simulink model "jgowans_fft_4chan"  (R2012b) (continued)
== system jgowans_fft_4chan/cross_multiplier1 == (continued)
      mask init:
      | pipeline_init(gcb,'latency',latency);
      settings: AttributesFormatString=Latency = %<latency>
      mask Display:
      | port_label('input', 1, 'd');
      | port_label('output', 1, 'q');
      | disp(['Z^-', num2str(latency)]);
  pipeline0_1_1_3 : Subsystem  AncestorBlock=casper_library_delays/pipeline  mask(pipeline){latency=fanout_latency}
      mask help:
      | An explicitly laid-out delay line to avoid mapping to SRL16s
      | for use in pipelining to help achieve timing closure.
      mask init:
      | pipeline_init(gcb,'latency',latency);
      settings: AttributesFormatString=Latency = %<latency>
      mask Display:
      | port_label('input', 1, 'd');
      | port_label('output', 1, 'q');
      | disp(['Z^-', num2str(latency)]);
  pipeline0_2_0_0 : Subsystem  AncestorBlock=casper_library_delays/pipeline  mask(pipeline){latency=fanout_latency}
      mask help:
      | An explicitly laid-out delay line to avoid mapping to SRL16s
      | for use in pipelining to help achieve timing closure.
      mask init:
      | pipeline_init(gcb,'latency',latency);
      settings: AttributesFormatString=Latency = %<latency>
      mask Display:
      | port_label('input', 1, 'd');
      | port_label('output', 1, 'q');
      | disp(['Z^-', num2str(latency)]);
  pipeline0_2_0_1 : Subsystem  AncestorBlock=casper_library_delays/pipeline  mask(pipeline){latency=fanout_latency}
      mask help:
      | An explicitly laid-out delay line to avoid mapping to SRL16s
      | for use in pipelining to help achieve timing closure.
      mask init:
      | pipeline_init(gcb,'latency',latency);
      settings: AttributesFormatString=Latency = %<latency>
      mask Display:
      | port_label('input', 1, 'd');
      | port_label('output', 1, 'q');
      | disp(['Z^-', num2str(latency)]);
  pipeline0_2_0_2 : Subsystem  AncestorBlock=casper_library_delays/pipeline  mask(pipeline){latency=fanout_latency}
      mask help:
      | An explicitly laid-out delay line to avoid mapping to SRL16s
      | for use in pipelining to help achieve timing closure.
      mask init:
      | pipeline_init(gcb,'latency',latency);
      settings: AttributesFormatString=Latency = %<latency>
      mask Display:
      | port_label('input', 1, 'd');
      | port_label('output', 1, 'q');
      | disp(['Z^-', num2str(latency)]);
  pipeline0_2_0_3 : Subsystem  AncestorBlock=casper_library_delays/pipeline  mask(pipeline){latency=fanout_latency}
      mask help:
      | An explicitly laid-out delay line to avoid mapping to SRL16s
      | for use in pipelining to help achieve timing closure.
      mask init:
      | pipeline_init(gcb,'latency',latency);
      settings: AttributesFormatString=Latency = %<latency>
      mask Display:
      | port_label('input', 1, 'd');
      | port_label('output', 1, 'q');
      | disp(['Z^-', num2str(latency)]);
  pipeline0_2_1_0 : Subsystem  AncestorBlock=casper_library_delays/pipeline  mask(pipeline){latency=fanout_latency}
      mask help:
      | An explicitly laid-out delay line to avoid mapping to SRL16s
      | for use in pipelining to help achieve timing closure.
      mask init:
      | pipeline_init(gcb,'latency',latency);
      settings: AttributesFormatString=Latency = %<latency>
      mask Display:
      | port_label('input', 1, 'd');
      | port_label('output', 1, 'q');
      | disp(['Z^-', num2str(latency)]);
  pipeline0_2_1_1 : Subsystem  AncestorBlock=casper_library_delays/pipeline  mask(pipeline){latency=fanout_latency}
      mask help:
      | An explicitly laid-out delay line to avoid mapping to SRL16s
      | for use in pipelining to help achieve timing closure.
      mask init:
      | pipeline_init(gcb,'latency',latency);
      settings: AttributesFormatString=Latency = %<latency>
      mask Display:
      | port_label('input', 1, 'd');
      | port_label('output', 1, 'q');
      | disp(['Z^-', num2str(latency)]);
  pipeline0_2_1_2 : Subsystem  AncestorBlock=casper_library_delays/pipeline  mask(pipeline){latency=fanout_latency}
      mask help:
      | An explicitly laid-out delay line to avoid mapping to SRL16s
      | for use in pipelining to help achieve timing closure.
      mask init:
      | pipeline_init(gcb,'latency',latency);
      settings: AttributesFormatString=Latency = %<latency>
      mask Display:
      | port_label('input', 1, 'd');
      | port_label('output', 1, 'q');
      | disp(['Z^-', num2str(latency)]);
  pipeline0_2_1_3 : Subsystem  AncestorBlock=casper_library_delays/pipeline  mask(pipeline){latency=fanout_latency}
      mask help:
      | An explicitly laid-out delay line to avoid mapping to SRL16s
      | for use in pipelining to help achieve timing closure.
      mask init:
      | pipeline_init(gcb,'latency',latency);
      settings: AttributesFormatString=Latency = %<latency>
      mask Display:
      | port_label('input', 1, 'd');
      | port_label('output', 1, 'q');
      | disp(['Z^-', num2str(latency)]);
  pipeline0_3_0_0 : Subsystem  AncestorBlock=casper_library_delays/pipeline  mask(pipeline){latency=fanout_latency}
      mask help:
      | An explicitly laid-out delay line to avoid mapping to SRL16s
      | for use in pipelining to help achieve timing closure.
      mask init:
      | pipeline_init(gcb,'latency',latency);
      settings: AttributesFormatString=Latency = %<latency>
      mask Display:
      | port_label('input', 1, 'd');
      | port_label('output', 1, 'q');
      | disp(['Z^-', num2str(latency)]);
  pipeline0_3_0_1 : Subsystem  AncestorBlock=casper_library_delays/pipeline  mask(pipeline){latency=fanout_latency}
      mask help:
      | An explicitly laid-out delay line to avoid mapping to SRL16s
      | for use in pipelining to help achieve timing closure.
      mask init:
      | pipeline_init(gcb,'latency',latency);
      settings: AttributesFormatString=Latency = %<latency>
      mask Display:
      | port_label('input', 1, 'd');
      | port_label('output', 1, 'q');
      | disp(['Z^-', num2str(latency)]);
  pipeline0_3_0_2 : Subsystem  AncestorBlock=casper_library_delays/pipeline  mask(pipeline){latency=fanout_latency}
      mask help:
      | An explicitly laid-out delay line to avoid mapping to SRL16s
      | for use in pipelining to help achieve timing closure.
      mask init:
      | pipeline_init(gcb,'latency',latency);
      settings: AttributesFormatString=Latency = %<latency>
      mask Display:
      | port_label('input', 1, 'd');
      | port_label('output', 1, 'q');
      | disp(['Z^-', num2str(latency)]);
  pipeline0_3_0_3 : Subsystem  AncestorBlock=casper_library_delays/pipeline  mask(pipeline){latency=fanout_latency}
      mask help:
      | An explicitly laid-out delay line to avoid mapping to SRL16s
      | for use in pipelining to help achieve timing closure.
      mask init:
      | pipeline_init(gcb,'latency',latency);
      settings: AttributesFormatString=Latency = %<latency>
      mask Display:
      | port_label('input', 1, 'd');
      | port_label('output', 1, 'q');
      | disp(['Z^-', num2str(latency)]);
  pipeline0_3_1_0 : Subsystem  AncestorBlock=casper_library_delays/pipeline  mask(pipeline){latency=fanout_latency}
      mask help:
      | An explicitly laid-out delay line to avoid mapping to SRL16s
      | for use in pipelining to help achieve timing closure.
      mask init:
      | pipeline_init(gcb,'latency',latency);
      settings: AttributesFormatString=Latency = %<latency>
      mask Display:
      | port_label('input', 1, 'd');
      | port_label('output', 1, 'q');
      | disp(['Z^-', num2str(latency)]);
  pipeline0_3_1_1 : Subsystem  AncestorBlock=casper_library_delays/pipeline  mask(pipeline){latency=fanout_latency}
      mask help:
      | An explicitly laid-out delay line to avoid mapping to SRL16s
      | for use in pipelining to help achieve timing closure.
      mask init:
      | pipeline_init(gcb,'latency',latency);
      settings: AttributesFormatString=Latency = %<latency>
      mask Display:
      | port_label('input', 1, 'd');
      | port_label('output', 1, 'q');
      | disp(['Z^-', num2str(latency)]);
  pipeline0_3_1_2 : Subsystem  AncestorBlock=casper_library_delays/pipeline  mask(pipeline){latency=fanout_latency}
      mask help:
      | An explicitly laid-out delay line to avoid mapping to SRL16s
      | for use in pipelining to help achieve timing closure.
      mask init:
      | pipeline_init(gcb,'latency',latency);
      settings: AttributesFormatString=Latency = %<latency>
      mask Display:
      | port_label('input', 1, 'd');
      | port_label('output', 1, 'q');
      | disp(['Z^-', num2str(latency)]);
  pipeline0_3_1_3 : Subsystem  AncestorBlock=casper_library_delays/pipeline  mask(pipeline){latency=fanout_latency}
      mask help:
      | An explicitly laid-out delay line to avoid mapping to SRL16s
      | for use in pipelining to help achieve timing closure.
      mask init:
      | pipeline_init(gcb,'latency',latency);
      settings: AttributesFormatString=Latency = %<latency>
      mask Display:
      | port_label('input', 1, 'd');
      | port_label('output', 1, 'q');
      | disp(['Z^-', num2str(latency)]);
  pipeline1_0_0_0 : Subsystem  AncestorBlock=casper_library_delays/pipeline  mask(pipeline){latency=fanout_latency}
      mask help:
      | An explicitly laid-out delay line to avoid mapping to SRL16s
      | for use in pipelining to help achieve timing closure.
      mask init:
      | pipeline_init(gcb,'latency',latency);
      settings: AttributesFormatString=Latency = %<latency>
      mask Display:
      | port_label('input', 1, 'd');
      | port_label('output', 1, 'q');
      | disp(['Z^-', num2str(latency)]);
  pipeline1_0_0_1 : Subsystem  AncestorBlock=casper_library_delays/pipeline  mask(pipeline){latency=fanout_latency}
      mask help:
      | An explicitly laid-out delay line to avoid mapping to SRL16s
      | for use in pipelining to help achieve timing closure.
      mask init:
      | pipeline_init(gcb,'latency',latency);
      settings: AttributesFormatString=Latency = %<latency>
      mask Display:
      | port_label('input', 1, 'd');
      | port_label('output', 1, 'q');
      | disp(['Z^-', num2str(latency)]);
  pipeline1_0_0_2 : Subsystem  AncestorBlock=casper_library_delays/pipeline  mask(pipeline){latency=fanout_latency}
      mask help:
      | An explicitly laid-out delay line to avoid mapping to SRL16s
      | for use in pipelining to help achieve timing closure.
      mask init:
      | pipeline_init(gcb,'latency',latency);
      settings: AttributesFormatString=Latency = %<latency>
      mask Display:
      | port_label('input', 1, 'd');
      | port_label('output', 1, 'q');
      | disp(['Z^-', num2str(latency)]);
  pipeline1_0_0_3 : Subsystem  AncestorBlock=casper_library_delays/pipeline  mask(pipeline){latency=fanout_latency}
      mask help:
      | An explicitly laid-out delay line to avoid mapping to SRL16s
      | for use in pipelining to help achieve timing closure.
      mask init:
      | pipeline_init(gcb,'latency',latency);
      settings: AttributesFormatString=Latency = %<latency>
      mask Display:
      | port_label('input', 1, 'd');
      | port_label('output', 1, 'q');
      | disp(['Z^-', num2str(latency)]);
  pipeline1_0_1_0 : Subsystem  AncestorBlock=casper_library_delays/pipeline  mask(pipeline){latency=fanout_latency}
      mask help:
      | An explicitly laid-out delay line to avoid mapping to SRL16s
      | for use in pipelining to help achieve timing closure.
      mask init:
      | pipeline_init(gcb,'latency',latency);
      settings: AttributesFormatString=Latency = %<latency>
      mask Display:
      | port_label('input', 1, 'd');
      | port_label('output', 1, 'q');
      | disp(['Z^-', num2str(latency)]);
  pipeline1_0_1_1 : Subsystem  AncestorBlock=casper_library_delays/pipeline  mask(pipeline){latency=fanout_latency}
      mask help:
      | An explicitly laid-out delay line to avoid mapping to SRL16s
      | for use in pipelining to help achieve timing closure.
      mask init:
      | pipeline_init(gcb,'latency',latency);
      settings: AttributesFormatString=Latency = %<latency>
      mask Display:
      | port_label('input', 1, 'd');
      | port_label('output', 1, 'q');
      | disp(['Z^-', num2str(latency)]);
  pipeline1_0_1_2 : Subsystem  AncestorBlock=casper_library_delays/pipeline  mask(pipeline){latency=fanout_latency}
      mask help:
      | An explicitly laid-out delay line to avoid mapping to SRL16s
      | for use in pipelining to help achieve timing closure.
      mask init:
      | pipeline_init(gcb,'latency',latency);
      settings: AttributesFormatString=Latency = %<latency>
      mask Display:
      | port_label('input', 1, 'd');
      | port_label('output', 1, 'q');
      | disp(['Z^-', num2str(latency)]);
  pipeline1_0_1_3 : Subsystem  AncestorBlock=casper_library_delays/pipeline  mask(pipeline){latency=fanout_latency}
      mask help:
      | An explicitly laid-out delay line to avoid mapping to SRL16s
      | for use in pipelining to help achieve timing closure.
      mask init:
      | pipeline_init(gcb,'latency',latency);
      settings: AttributesFormatString=Latency = %<latency>
      mask Display:
      | port_label('input', 1, 'd');
      | port_label('output', 1, 'q');
      | disp(['Z^-', num2str(latency)]);
  pipeline1_1_0_0 : Subsystem  AncestorBlock=casper_library_delays/pipeline  mask(pipeline){latency=fanout_latency}
      mask help:
      | An explicitly laid-out delay line to avoid mapping to SRL16s
      | for use in pipelining to help achieve timing closure.
      mask init:
      | pipeline_init(gcb,'latency',latency);
      settings: AttributesFormatString=Latency = %<latency>
      mask Display:
      | port_label('input', 1, 'd');
      | port_label('output', 1, 'q');
      | disp(['Z^-', num2str(latency)]);
  pipeline1_1_0_1 : Subsystem  AncestorBlock=casper_library_delays/pipeline  mask(pipeline){latency=fanout_latency}
      mask help:
      | An explicitly laid-out delay line to avoid mapping to SRL16s
      | for use in pipelining to help achieve timing closure.
      mask init:
      | pipeline_init(gcb,'latency',latency);
      settings: AttributesFormatString=Latency = %<latency>
      mask Display:
      | port_label('input', 1, 'd');
      | port_label('output', 1, 'q');
      | disp(['Z^-', num2str(latency)]);
  pipeline1_1_0_2 : Subsystem  AncestorBlock=casper_library_delays/pipeline  mask(pipeline){latency=fanout_latency}
      mask help:
      | An explicitly laid-out delay line to avoid mapping to SRL16s
      | for use in pipelining to help achieve timing closure.
      mask init:
      | pipeline_init(gcb,'latency',latency);
      settings: AttributesFormatString=Latency = %<latency>
      mask Display:
      | port_label('input', 1, 'd');
      | port_label('output', 1, 'q');
      | disp(['Z^-', num2str(latency)]);
  pipeline1_1_0_3 : Subsystem  AncestorBlock=casper_library_delays/pipeline  mask(pipeline){latency=fanout_latency}
      mask help:
      | An explicitly laid-out delay line to avoid mapping to SRL16s
      | for use in pipelining to help achieve timing closure.
      mask init:
      | pipeline_init(gcb,'latency',latency);
      settings: AttributesFormatString=Latency = %<latency>
      mask Display:
      | port_label('input', 1, 'd');
      | port_label('output', 1, 'q');
      | disp(['Z^-', num2str(latency)]);
  pipeline1_1_1_0 : Subsystem  AncestorBlock=casper_library_delays/pipeline  mask(pipeline){latency=fanout_latency}
      mask help:
      | An explicitly laid-out delay line to avoid mapping to SRL16s
      | for use in pipelining to help achieve timing closure.
      mask init:
      | pipeline_init(gcb,'latency',latency);
      settings: AttributesFormatString=Latency = %<latency>
      mask Display:
      | port_label('input', 1, 'd');
      | port_label('output', 1, 'q');
      | disp(['Z^-', num2str(latency)]);
  pipeline1_1_1_1 : Subsystem  AncestorBlock=casper_library_delays/pipeline  mask(pipeline){latency=fanout_latency}
      mask help:
      | An explicitly laid-out delay line to avoid mapping to SRL16s
      | for use in pipelining to help achieve timing closure.
      mask init:
      | pipeline_init(gcb,'latency',latency);
      settings: AttributesFormatString=Latency = %<latency>
      mask Display:
      | port_label('input', 1, 'd');
      | port_label('output', 1, 'q');
      | disp(['Z^-', num2str(latency)]);
  pipeline1_1_1_2 : Subsystem  AncestorBlock=casper_library_delays/pipeline  mask(pipeline){latency=fanout_latency}
      mask help:
      | An explicitly laid-out delay line to avoid mapping to SRL16s
      | for use in pipelining to help achieve timing closure.
      mask init:
      | pipeline_init(gcb,'latency',latency);
      settings: AttributesFormatString=Latency = %<latency>
      mask Display:
      | port_label('input', 1, 'd');
      | port_label('output', 1, 'q');
      | disp(['Z^-', num2str(latency)]);
  pipeline1_1_1_3 : Subsystem  AncestorBlock=casper_library_delays/pipeline  mask(pipeline){latency=fanout_latency}
      mask help:
      | An explicitly laid-out delay line to avoid mapping to SRL16s
      | for use in pipelining to help achieve timing closure.
      mask init:
      | pipeline_init(gcb,'latency',latency);
      settings: AttributesFormatString=Latency = %<latency>
      mask Display:
      | port_label('input', 1, 'd');
      | port_label('output', 1, 'q');
      | disp(['Z^-', num2str(latency)]);
  pipeline1_2_0_0 : Subsystem  AncestorBlock=casper_library_delays/pipeline  mask(pipeline){latency=fanout_latency}
      mask help:
      | An explicitly laid-out delay line to avoid mapping to SRL16s
      | for use in pipelining to help achieve timing closure.
      mask init:
      | pipeline_init(gcb,'latency',latency);
      settings: AttributesFormatString=Latency = %<latency>
      mask Display:
      | port_label('input', 1, 'd');
      | port_label('output', 1, 'q');
      | disp(['Z^-', num2str(latency)]);
  pipeline1_2_0_1 : Subsystem  AncestorBlock=casper_library_delays/pipeline  mask(pipeline){latency=fanout_latency}
      mask help:
      | An explicitly laid-out delay line to avoid mapping to SRL16s
      | for use in pipelining to help achieve timing closure.
      mask init:
      | pipeline_init(gcb,'latency',latency);
      settings: AttributesFormatString=Latency = %<latency>
      mask Display:
      | port_label('input', 1, 'd');
      | port_label('output', 1, 'q');
      | disp(['Z^-', num2str(latency)]);
  pipeline1_2_0_2 : Subsystem  AncestorBlock=casper_library_delays/pipeline  mask(pipeline){latency=fanout_latency}
      mask help:
      | An explicitly laid-out delay line to avoid mapping to SRL16s
      | for use in pipelining to help achieve timing closure.
      mask init:
      | pipeline_init(gcb,'latency',latency);
      settings: AttributesFormatString=Latency = %<latency>
      mask Display:
      | port_label('input', 1, 'd');
      | port_label('output', 1, 'q');
      | disp(['Z^-', num2str(latency)]);
  pipeline1_2_0_3 : Subsystem  AncestorBlock=casper_library_delays/pipeline  mask(pipeline){latency=fanout_latency}
      mask help:
      | An explicitly laid-out delay line to avoid mapping to SRL16s
      | for use in pipelining to help achieve timing closure.
      mask init:
      | pipeline_init(gcb,'latency',latency);
      settings: AttributesFormatString=Latency = %<latency>
      mask Display:
      | port_label('input', 1, 'd');
      | port_label('output', 1, 'q');
      | disp(['Z^-', num2str(latency)]);
  pipeline1_2_1_0 : Subsystem  AncestorBlock=casper_library_delays/pipeline  mask(pipeline){latency=fanout_latency}
      mask help:
      | An explicitly laid-out delay line to avoid mapping to SRL16s
      | for use in pipelining to help achieve timing closure.
      mask init:
      | pipeline_init(gcb,'latency',latency);
      settings: AttributesFormatString=Latency = %<latency>
      mask Display:
      | port_label('input', 1, 'd');
      | port_label('output', 1, 'q');
      | disp(['Z^-', num2str(latency)]);
  pipeline1_2_1_1 : Subsystem  AncestorBlock=casper_library_delays/pipeline  mask(pipeline){latency=fanout_latency}
      mask help:
      | An explicitly laid-out delay line to avoid mapping to SRL16s
      | for use in pipelining to help achieve timing closure.
      mask init:
      | pipeline_init(gcb,'latency',latency);
      settings: AttributesFormatString=Latency = %<latency>
      mask Display:
      | port_label('input', 1, 'd');
      | port_label('output', 1, 'q');
      | disp(['Z^-', num2str(latency)]);
  pipeline1_2_1_2 : Subsystem  AncestorBlock=casper_library_delays/pipeline  mask(pipeline){latency=fanout_latency}
      mask help:
      | An explicitly laid-out delay line to avoid mapping to SRL16s
      | for use in pipelining to help achieve timing closure.
      mask init:
      | pipeline_init(gcb,'latency',latency);
      settings: AttributesFormatString=Latency = %<latency>
      mask Display:
      | port_label('input', 1, 'd');
      | port_label('output', 1, 'q');
      | disp(['Z^-', num2str(latency)]);
  pipeline1_2_1_3 : Subsystem  AncestorBlock=casper_library_delays/pipeline  mask(pipeline){latency=fanout_latency}
      mask help:
      | An explicitly laid-out delay line to avoid mapping to SRL16s
      | for use in pipelining to help achieve timing closure.
      mask init:
      | pipeline_init(gcb,'latency',latency);
      settings: AttributesFormatString=Latency = %<latency>
      mask Display:
      | port_label('input', 1, 'd');
      | port_label('output', 1, 'q');
      | disp(['Z^-', num2str(latency)]);
  pipeline2_0_0_0 : Subsystem  AncestorBlock=casper_library_delays/pipeline  mask(pipeline){latency=fanout_latency}
      mask help:
      | An explicitly laid-out delay line to avoid mapping to SRL16s
      | for use in pipelining to help achieve timing closure.
      mask init:
      | pipeline_init(gcb,'latency',latency);
      settings: AttributesFormatString=Latency = %<latency>
      mask Display:
      | port_label('input', 1, 'd');
      | port_label('output', 1, 'q');
      | disp(['Z^-', num2str(latency)]);
  pipeline2_0_0_1 : Subsystem  AncestorBlock=casper_library_delays/pipeline  mask(pipeline){latency=fanout_latency}
      mask help:
      | An explicitly laid-out delay line to avoid mapping to SRL16s
      | for use in pipelining to help achieve timing closure.
      mask init:
      | pipeline_init(gcb,'latency',latency);
      settings: AttributesFormatString=Latency = %<latency>
      mask Display:
      | port_label('input', 1, 'd');
      | port_label('output', 1, 'q');
      | disp(['Z^-', num2str(latency)]);
  pipeline2_0_0_2 : Subsystem  AncestorBlock=casper_library_delays/pipeline  mask(pipeline){latency=fanout_latency}
      mask help:
      | An explicitly laid-out delay line to avoid mapping to SRL16s
      | for use in pipelining to help achieve timing closure.
      mask init:
      | pipeline_init(gcb,'latency',latency);
      settings: AttributesFormatString=Latency = %<latency>
      mask Display:
      | port_label('input', 1, 'd');
      | port_label('output', 1, 'q');
      | disp(['Z^-', num2str(latency)]);
  pipeline2_0_0_3 : Subsystem  AncestorBlock=casper_library_delays/pipeline  mask(pipeline){latency=fanout_latency}
      mask help:
      | An explicitly laid-out delay line to avoid mapping to SRL16s
      | for use in pipelining to help achieve timing closure.
      mask init:
      | pipeline_init(gcb,'latency',latency);
      settings: AttributesFormatString=Latency = %<latency>
      mask Display:
      | port_label('input', 1, 'd');
      | port_label('output', 1, 'q');
      | disp(['Z^-', num2str(latency)]);
  pipeline2_0_1_0 : Subsystem  AncestorBlock=casper_library_delays/pipeline  mask(pipeline){latency=fanout_latency}
      mask help:
      | An explicitly laid-out delay line to avoid mapping to SRL16s
      | for use in pipelining to help achieve timing closure.
      mask init:
      | pipeline_init(gcb,'latency',latency);
      settings: AttributesFormatString=Latency = %<latency>
      mask Display:
      | port_label('input', 1, 'd');
      | port_label('output', 1, 'q');
      | disp(['Z^-', num2str(latency)]);
  pipeline2_0_1_1 : Subsystem  AncestorBlock=casper_library_delays/pipeline  mask(pipeline){latency=fanout_latency}
      mask help:
      | An explicitly laid-out delay line to avoid mapping to SRL16s
      | for use in pipelining to help achieve timing closure.
      mask init:
      | pipeline_init(gcb,'latency',latency);
      settings: AttributesFormatString=Latency = %<latency>
      mask Display:
      | port_label('input', 1, 'd');
      | port_label('output', 1, 'q');
      | disp(['Z^-', num2str(latency)]);
  pipeline2_0_1_2 : Subsystem  AncestorBlock=casper_library_delays/pipeline  mask(pipeline){latency=fanout_latency}
      mask help:
      | An explicitly laid-out delay line to avoid mapping to SRL16s
      | for use in pipelining to help achieve timing closure.
      mask init:
      | pipeline_init(gcb,'latency',latency);
      settings: AttributesFormatString=Latency = %<latency>
      mask Display:
      | port_label('input', 1, 'd');
      | port_label('output', 1, 'q');
      | disp(['Z^-', num2str(latency)]);
  pipeline2_0_1_3 : Subsystem  AncestorBlock=casper_library_delays/pipeline  mask(pipeline){latency=fanout_latency}
      mask help:
      | An explicitly laid-out delay line to avoid mapping to SRL16s
      | for use in pipelining to help achieve timing closure.
      mask init:
      | pipeline_init(gcb,'latency',latency);
      settings: AttributesFormatString=Latency = %<latency>
      mask Display:
      | port_label('input', 1, 'd');
      | port_label('output', 1, 'q');
      | disp(['Z^-', num2str(latency)]);
  pipeline2_1_0_0 : Subsystem  AncestorBlock=casper_library_delays/pipeline  mask(pipeline){latency=fanout_latency}
      mask help:
      | An explicitly laid-out delay line to avoid mapping to SRL16s
      | for use in pipelining to help achieve timing closure.
      mask init:
      | pipeline_init(gcb,'latency',latency);
      settings: AttributesFormatString=Latency = %<latency>
      mask Display:
      | port_label('input', 1, 'd');
      | port_label('output', 1, 'q');
      | disp(['Z^-', num2str(latency)]);
  pipeline2_1_0_1 : Subsystem  AncestorBlock=casper_library_delays/pipeline  mask(pipeline){latency=fanout_latency}
      mask help:
      | An explicitly laid-out delay line to avoid mapping to SRL16s
      | for use in pipelining to help achieve timing closure.
      mask init:
      | pipeline_init(gcb,'latency',latency);
      settings: AttributesFormatString=Latency = %<latency>
      mask Display:
      | port_label('input', 1, 'd');
      | port_label('output', 1, 'q');
      | disp(['Z^-', num2str(latency)]);
  pipeline2_1_0_2 : Subsystem  AncestorBlock=casper_library_delays/pipeline  mask(pipeline){latency=fanout_latency}
      mask help:
      | An explicitly laid-out delay line to avoid mapping to SRL16s
      | for use in pipelining to help achieve timing closure.
      mask init:
      | pipeline_init(gcb,'latency',latency);
      settings: AttributesFormatString=Latency = %<latency>
      mask Display:
      | port_label('input', 1, 'd');
      | port_label('output', 1, 'q');
      | disp(['Z^-', num2str(latency)]);
  pipeline2_1_0_3 : Subsystem  AncestorBlock=casper_library_delays/pipeline  mask(pipeline){latency=fanout_latency}
      mask help:
      | An explicitly laid-out delay line to avoid mapping to SRL16s
      | for use in pipelining to help achieve timing closure.
      mask init:
      | pipeline_init(gcb,'latency',latency);
      settings: AttributesFormatString=Latency = %<latency>
      mask Display:
      | port_label('input', 1, 'd');
      | port_label('output', 1, 'q');
      | disp(['Z^-', num2str(latency)]);
  pipeline2_1_1_0 : Subsystem  AncestorBlock=casper_library_delays/pipeline  mask(pipeline){latency=fanout_latency}
      mask help:
      | An explicitly laid-out delay line to avoid mapping to SRL16s
      | for use in pipelining to help achieve timing closure.
      mask init:
      | pipeline_init(gcb,'latency',latency);
      settings: AttributesFormatString=Latency = %<latency>
      mask Display:
      | port_label('input', 1, 'd');
      | port_label('output', 1, 'q');
      | disp(['Z^-', num2str(latency)]);
  pipeline2_1_1_1 : Subsystem  AncestorBlock=casper_library_delays/pipeline  mask(pipeline){latency=fanout_latency}
      mask help:
      | An explicitly laid-out delay line to avoid mapping to SRL16s
      | for use in pipelining to help achieve timing closure.
      mask init:
      | pipeline_init(gcb,'latency',latency);
      settings: AttributesFormatString=Latency = %<latency>
      mask Display:
      | port_label('input', 1, 'd');
      | port_label('output', 1, 'q');
      | disp(['Z^-', num2str(latency)]);
  pipeline2_1_1_2 : Subsystem  AncestorBlock=casper_library_delays/pipeline  mask(pipeline){latency=fanout_latency}
      mask help:
      | An explicitly laid-out delay line to avoid mapping to SRL16s
      | for use in pipelining to help achieve timing closure.
      mask init:
      | pipeline_init(gcb,'latency',latency);
      settings: AttributesFormatString=Latency = %<latency>
      mask Display:
      | port_label('input', 1, 'd');
      | port_label('output', 1, 'q');
      | disp(['Z^-', num2str(latency)]);
  pipeline2_1_1_3 : Subsystem  AncestorBlock=casper_library_delays/pipeline  mask(pipeline){latency=fanout_latency}
      mask help:
      | An explicitly laid-out delay line to avoid mapping to SRL16s
      | for use in pipelining to help achieve timing closure.
      mask init:
      | pipeline_init(gcb,'latency',latency);
      settings: AttributesFormatString=Latency = %<latency>
      mask Display:
      | port_label('input', 1, 'd');
      | port_label('output', 1, 'q');
      | disp(['Z^-', num2str(latency)]);
  pipeline3_0_0_0 : Subsystem  AncestorBlock=casper_library_delays/pipeline  mask(pipeline){latency=fanout_latency}
      mask help:
      | An explicitly laid-out delay line to avoid mapping to SRL16s
      | for use in pipelining to help achieve timing closure.
      mask init:
      | pipeline_init(gcb,'latency',latency);
      settings: AttributesFormatString=Latency = %<latency>
      mask Display:
      | port_label('input', 1, 'd');
      | port_label('output', 1, 'q');
      | disp(['Z^-', num2str(latency)]);
  pipeline3_0_0_1 : Subsystem  AncestorBlock=casper_library_delays/pipeline  mask(pipeline){latency=fanout_latency}
      mask help:
      | An explicitly laid-out delay line to avoid mapping to SRL16s
      | for use in pipelining to help achieve timing closure.
      mask init:
      | pipeline_init(gcb,'latency',latency);
      settings: AttributesFormatString=Latency = %<latency>
      mask Display:
      | port_label('input', 1, 'd');
      | port_label('output', 1, 'q');
      | disp(['Z^-', num2str(latency)]);
  pipeline3_0_0_2 : Subsystem  AncestorBlock=casper_library_delays/pipeline  mask(pipeline){latency=fanout_latency}
      mask help:
      | An explicitly laid-out delay line to avoid mapping to SRL16s
      | for use in pipelining to help achieve timing closure.
      mask init:
      | pipeline_init(gcb,'latency',latency);
      settings: AttributesFormatString=Latency = %<latency>
      mask Display:
      | port_label('input', 1, 'd');
      | port_label('output', 1, 'q');
      | disp(['Z^-', num2str(latency)]);
  pipeline3_0_0_3 : Subsystem  AncestorBlock=casper_library_delays/pipeline  mask(pipeline){latency=fanout_latency}
      mask help:
      | An explicitly laid-out delay line to avoid mapping to SRL16s
      | for use in pipelining to help achieve timing closure.
      mask init:
      | pipeline_init(gcb,'latency',latency);
      settings: AttributesFormatString=Latency = %<latency>
      mask Display:
      | port_label('input', 1, 'd');
      | port_label('output', 1, 'q');
      | disp(['Z^-', num2str(latency)]);
  pipeline3_0_1_0 : Subsystem  AncestorBlock=casper_library_delays/pipeline  mask(pipeline){latency=fanout_latency}
      mask help:
      | An explicitly laid-out delay line to avoid mapping to SRL16s
      | for use in pipelining to help achieve timing closure.
      mask init:
      | pipeline_init(gcb,'latency',latency);
      settings: AttributesFormatString=Latency = %<latency>
      mask Display:
      | port_label('input', 1, 'd');
      | port_label('output', 1, 'q');
      | disp(['Z^-', num2str(latency)]);
  pipeline3_0_1_1 : Subsystem  AncestorBlock=casper_library_delays/pipeline  mask(pipeline){latency=fanout_latency}
      mask help:
      | An explicitly laid-out delay line to avoid mapping to SRL16s
      | for use in pipelining to help achieve timing closure.
      mask init:
      | pipeline_init(gcb,'latency',latency);
      settings: AttributesFormatString=Latency = %<latency>
      mask Display:
      | port_label('input', 1, 'd');
      | port_label('output', 1, 'q');
      | disp(['Z^-', num2str(latency)]);
  pipeline3_0_1_2 : Subsystem  AncestorBlock=casper_library_delays/pipeline  mask(pipeline){latency=fanout_latency}
      mask help:
      | An explicitly laid-out delay line to avoid mapping to SRL16s
      | for use in pipelining to help achieve timing closure.
      mask init:
      | pipeline_init(gcb,'latency',latency);
      settings: AttributesFormatString=Latency = %<latency>
      mask Display:
      | port_label('input', 1, 'd');
      | port_label('output', 1, 'q');
      | disp(['Z^-', num2str(latency)]);
  pipeline3_0_1_3 : Subsystem  AncestorBlock=casper_library_delays/pipeline  mask(pipeline){latency=fanout_latency}
      mask help:
      | An explicitly laid-out delay line to avoid mapping to SRL16s
      | for use in pipelining to help achieve timing closure.
      mask init:
      | pipeline_init(gcb,'latency',latency);
      settings: AttributesFormatString=Latency = %<latency>
      mask Display:
      | port_label('input', 1, 'd');
      | port_label('output', 1, 'q');
      | disp(['Z^-', num2str(latency)]);
  ri_to_c0_0_0 : casper_library_misc/ri_to_c
  ri_to_c0_0_1 : casper_library_misc/ri_to_c
  ri_to_c0_1_0 : casper_library_misc/ri_to_c
  ri_to_c0_1_1 : casper_library_misc/ri_to_c
  ri_to_c0_2_0 : casper_library_misc/ri_to_c
  ri_to_c0_2_1 : casper_library_misc/ri_to_c
  ri_to_c0_3_0 : casper_library_misc/ri_to_c
  ri_to_c0_3_1 : casper_library_misc/ri_to_c
  ri_to_c1_0_0 : casper_library_misc/ri_to_c
  ri_to_c1_0_1 : casper_library_misc/ri_to_c
  ri_to_c1_1_0 : casper_library_misc/ri_to_c
  ri_to_c1_1_1 : casper_library_misc/ri_to_c
  ri_to_c1_2_0 : casper_library_misc/ri_to_c
  ri_to_c1_2_1 : casper_library_misc/ri_to_c
  ri_to_c2_0_0 : casper_library_misc/ri_to_c
  ri_to_c2_0_1 : casper_library_misc/ri_to_c
  ri_to_c2_1_0 : casper_library_misc/ri_to_c
  ri_to_c2_1_1 : casper_library_misc/ri_to_c
  ri_to_c3_0_0 : casper_library_misc/ri_to_c
  ri_to_c3_0_1 : casper_library_misc/ri_to_c
  sync_delay : xbsIndex_r4/Delay  rst=off  infoeditControl=Selection of Reset will increase slice count due to use of real FFs and instead of SRLs  en=off  latency=mult_latency+add_latency+conv_latency+fanout_latency  dbl_ovrd=off  reg_retiming=off  block_type=delay
  unpack0 : Subsystem  AncestorBlock=casper_library_flow_control/bus_expand  mask(bus_expand){mode=divisions of equal size, outputNum=aggregation, outputWidth=bit_width_in*2, outputBinaryPt=0, outputArithmeticType=0, show_format=off, outputToWorkspace=off, variablePrefix=out, outputToModelAsWell=on}
      mask prompts: outputNum "Number of outputs:"; outputWidth "Output width:"; outputBinaryPt "Output binary point position:"; outputArithmeticType "Output arithmetic type (ufix=0, fix=1, bool=2):"; show_format "Print format string?"; outputToWorkspace "Output to workspace?"; variablePrefix "Variable name prefix:"; outputToModelAsWell "Output to model as well?"
      mask help:
      | Expands a 'bus' made using a bus_create or Xilinx
      | concat block.
      | The input will be divided into the specified number
      | of outputs with the specified arithmetic types.
      | Divisions are made from most significant bit.
      mask init: same as jgowans_fft_4chan/bus_expand
      settings: AttributesFormatString=2 outputs
      mask TypeOptions mode: divisions of equal size | divisions of arbitrary size
      mask Callback mode: bus_expand_callback();
      mask Callback outputWidth: bus_expand_callback();
      mask Callback outputToWorkspace: bus_expand_callback();
  unpack1 : Subsystem  AncestorBlock=casper_library_flow_control/bus_expand  mask(bus_expand){mode=divisions of equal size, outputNum=aggregation, outputWidth=bit_width_in*2, outputBinaryPt=0, outputArithmeticType=0, show_format=off, outputToWorkspace=off, variablePrefix=out, outputToModelAsWell=on}
      mask prompts: outputNum "Number of outputs:"; outputWidth "Output width:"; outputBinaryPt "Output binary point position:"; outputArithmeticType "Output arithmetic type (ufix=0, fix=1, bool=2):"; show_format "Print format string?"; outputToWorkspace "Output to workspace?"; variablePrefix "Variable name prefix:"; outputToModelAsWell "Output to model as well?"
      mask help:
      | Expands a 'bus' made using a bus_create or Xilinx
      | concat block.
      | The input will be divided into the specified number
      | of outputs with the specified arithmetic types.
      | Divisions are made from most significant bit.
      mask init: same as jgowans_fft_4chan/bus_expand
      settings: AttributesFormatString=2 outputs
      mask TypeOptions mode: divisions of equal size | divisions of arbitrary size
      mask Callback mode: bus_expand_callback();
      mask Callback outputWidth: bus_expand_callback();
      mask Callback outputToWorkspace: bus_expand_callback();
  unpack2 : Subsystem  AncestorBlock=casper_library_flow_control/bus_expand  mask(bus_expand){mode=divisions of equal size, outputNum=aggregation, outputWidth=bit_width_in*2, outputBinaryPt=0, outputArithmeticType=0, show_format=off, outputToWorkspace=off, variablePrefix=out, outputToModelAsWell=on}
      mask prompts: outputNum "Number of outputs:"; outputWidth "Output width:"; outputBinaryPt "Output binary point position:"; outputArithmeticType "Output arithmetic type (ufix=0, fix=1, bool=2):"; show_format "Print format string?"; outputToWorkspace "Output to workspace?"; variablePrefix "Variable name prefix:"; outputToModelAsWell "Output to model as well?"
      mask help:
      | Expands a 'bus' made using a bus_create or Xilinx
      | concat block.
      | The input will be divided into the specified number
      | of outputs with the specified arithmetic types.
      | Divisions are made from most significant bit.
      mask init: same as jgowans_fft_4chan/bus_expand
      settings: AttributesFormatString=2 outputs
      mask TypeOptions mode: divisions of equal size | divisions of arbitrary size
      mask Callback mode: bus_expand_callback();
      mask Callback outputWidth: bus_expand_callback();
      mask Callback outputToWorkspace: bus_expand_callback();
  unpack3 : Subsystem  AncestorBlock=casper_library_flow_control/bus_expand  mask(bus_expand){mode=divisions of equal size, outputNum=aggregation, outputWidth=bit_width_in*2, outputBinaryPt=0, outputArithmeticType=0, show_format=off, outputToWorkspace=off, variablePrefix=out, outputToModelAsWell=on}
      mask prompts: outputNum "Number of outputs:"; outputWidth "Output width:"; outputBinaryPt "Output binary point position:"; outputArithmeticType "Output arithmetic type (ufix=0, fix=1, bool=2):"; show_format "Print format string?"; outputToWorkspace "Output to workspace?"; variablePrefix "Variable name prefix:"; outputToModelAsWell "Output to model as well?"
      mask help:
      | Expands a 'bus' made using a bus_create or Xilinx
      | concat block.
      | The input will be divided into the specified number
      | of outputs with the specified arithmetic types.
      | Divisions are made from most significant bit.
      mask init: same as jgowans_fft_4chan/bus_expand
      settings: AttributesFormatString=2 outputs
      mask TypeOptions mode: divisions of equal size | divisions of arbitrary size
      mask Callback mode: bus_expand_callback();
      mask Callback outputWidth: bus_expand_callback();
      mask Callback outputToWorkspace: bus_expand_callback();
  sync_out : Outport
  din0_x_din0* : Outport  Port=2
  din0_x_din1* : Outport  Port=3
  din0_x_din2* : Outport  Port=4
  din0_x_din3* : Outport  Port=5
  din1_x_din1* : Outport  Port=6
  din1_x_din2* : Outport  Port=7
  din1_x_din3* : Outport  Port=8
  din2_x_din2* : Outport  Port=9
  din2_x_din3* : Outport  Port=10
  din3_x_din3* : Outport  Port=11
  wires:
  sync_in/1 -> sync_delay/1
  sync_delay/1 -> sync_out/1
  din0/1 -> unpack0/1(bus_in)
  unpack0/1(msb_out2) -> c_to_ri0_0/1
  unpack0/2(lsb_out1) -> c_to_ri0_1/1
  din1/1 -> unpack1/1(bus_in)
  unpack1/1(msb_out2) -> c_to_ri1_0/1
  unpack1/2(lsb_out1) -> c_to_ri1_1/1
  din2/1 -> unpack2/1(bus_in)
  unpack2/1(msb_out2) -> c_to_ri2_0/1
  unpack2/2(lsb_out1) -> c_to_ri2_1/1
  din3/1 -> unpack3/1(bus_in)
  unpack3/1(msb_out2) -> c_to_ri3_0/1
  unpack3/2(lsb_out1) -> c_to_ri3_1/1
  c_to_ri0_0/1 -> pipeline0_0_0_0/1(d), pipeline0_0_0_2/1(d), pipeline0_1_0_0/1(d), pipeline0_2_0_0/1(d), pipeline0_3_0_0/1(d)
  pipeline0_0_0_0/1(q) -> cmult0_0_0/1
  c_to_ri0_0/2 -> pipeline0_0_0_1/1(d), pipeline0_0_0_3/1(d), pipeline0_1_0_1/1(d), pipeline0_2_0_1/1(d), pipeline0_3_0_1/1(d)
  pipeline0_0_0_1/1(q) -> cmult0_0_0/2
  pipeline0_0_0_2/1(q) -> cmult0_0_0/3
  pipeline0_0_0_3/1(q) -> cmult0_0_0/4
  cmult0_0_0/1 -> cvrt0_0_0_real/1(din)
  cmult0_0_0/2 -> cvrt0_0_0_imag/1(din)
  cvrt0_0_0_real/1(dout) -> ri_to_c0_0_0/1
  cvrt0_0_0_imag/1(dout) -> ri_to_c0_0_0/2
  ri_to_c0_0_0/1 -> pack0_0/1(in1)
  c_to_ri0_1/1 -> pipeline0_0_1_0/1(d), pipeline0_0_1_2/1(d), pipeline0_1_1_0/1(d), pipeline0_2_1_0/1(d), pipeline0_3_1_0/1(d)
  pipeline0_0_1_0/1(q) -> cmult0_0_1/1
  c_to_ri0_1/2 -> pipeline0_0_1_1/1(d), pipeline0_0_1_3/1(d), pipeline0_1_1_1/1(d), pipeline0_2_1_1/1(d), pipeline0_3_1_1/1(d)
  pipeline0_0_1_1/1(q) -> cmult0_0_1/2
  pipeline0_0_1_2/1(q) -> cmult0_0_1/3
  pipeline0_0_1_3/1(q) -> cmult0_0_1/4
  cmult0_0_1/1 -> cvrt0_0_1_real/1(din)
  cmult0_0_1/2 -> cvrt0_0_1_imag/1(din)
  cvrt0_0_1_real/1(dout) -> ri_to_c0_0_1/1
  cvrt0_0_1_imag/1(dout) -> ri_to_c0_0_1/2
  ri_to_c0_0_1/1 -> pack0_0/2(in2)
  pack0_0/1(bus_out) -> din0_x_din0*/1
  pipeline0_1_0_0/1(q) -> cmult0_1_0/1
  pipeline0_1_0_1/1(q) -> cmult0_1_0/2
  c_to_ri1_0/1 -> pipeline0_1_0_2/1(d), pipeline1_0_0_0/1(d), pipeline1_0_0_2/1(d), pipeline1_1_0_0/1(d), pipeline1_2_0_0/1(d)
  pipeline0_1_0_2/1(q) -> cmult0_1_0/3
  c_to_ri1_0/2 -> pipeline0_1_0_3/1(d), pipeline1_0_0_1/1(d), pipeline1_0_0_3/1(d), pipeline1_1_0_1/1(d), pipeline1_2_0_1/1(d)
  pipeline0_1_0_3/1(q) -> cmult0_1_0/4
  cmult0_1_0/1 -> cvrt0_1_0_real/1(din)
  cmult0_1_0/2 -> cvrt0_1_0_imag/1(din)
  cvrt0_1_0_real/1(dout) -> ri_to_c0_1_0/1
  cvrt0_1_0_imag/1(dout) -> ri_to_c0_1_0/2
  ri_to_c0_1_0/1 -> pack0_1/1(in1)
  pipeline0_1_1_0/1(q) -> cmult0_1_1/1
  pipeline0_1_1_1/1(q) -> cmult0_1_1/2
  c_to_ri1_1/1 -> pipeline0_1_1_2/1(d), pipeline1_0_1_0/1(d), pipeline1_0_1_2/1(d), pipeline1_1_1_0/1(d), pipeline1_2_1_0/1(d)
  pipeline0_1_1_2/1(q) -> cmult0_1_1/3
  c_to_ri1_1/2 -> pipeline0_1_1_3/1(d), pipeline1_0_1_1/1(d), pipeline1_0_1_3/1(d), pipeline1_1_1_1/1(d), pipeline1_2_1_1/1(d)
  pipeline0_1_1_3/1(q) -> cmult0_1_1/4
  cmult0_1_1/1 -> cvrt0_1_1_real/1(din)
  cmult0_1_1/2 -> cvrt0_1_1_imag/1(din)
  cvrt0_1_1_real/1(dout) -> ri_to_c0_1_1/1
  cvrt0_1_1_imag/1(dout) -> ri_to_c0_1_1/2
  ri_to_c0_1_1/1 -> pack0_1/2(in2)
  pack0_1/1(bus_out) -> din0_x_din1*/1
  pipeline0_2_0_0/1(q) -> cmult0_2_0/1
  pipeline0_2_0_1/1(q) -> cmult0_2_0/2
  c_to_ri2_0/1 -> pipeline0_2_0_2/1(d), pipeline1_1_0_2/1(d), pipeline2_0_0_0/1(d), pipeline2_0_0_2/1(d), pipeline2_1_0_0/1(d)
  pipeline0_2_0_2/1(q) -> cmult0_2_0/3
  c_to_ri2_0/2 -> pipeline0_2_0_3/1(d), pipeline1_1_0_3/1(d), pipeline2_0_0_1/1(d), pipeline2_0_0_3/1(d), pipeline2_1_0_1/1(d)
  pipeline0_2_0_3/1(q) -> cmult0_2_0/4
  cmult0_2_0/1 -> cvrt0_2_0_real/1(din)
  cmult0_2_0/2 -> cvrt0_2_0_imag/1(din)
  cvrt0_2_0_real/1(dout) -> ri_to_c0_2_0/1
  cvrt0_2_0_imag/1(dout) -> ri_to_c0_2_0/2
  ri_to_c0_2_0/1 -> pack0_2/1(in1)
  pipeline0_2_1_0/1(q) -> cmult0_2_1/1
  pipeline0_2_1_1/1(q) -> cmult0_2_1/2
  c_to_ri2_1/1 -> pipeline0_2_1_2/1(d), pipeline1_1_1_2/1(d), pipeline2_0_1_0/1(d), pipeline2_0_1_2/1(d), pipeline2_1_1_0/1(d)
  pipeline0_2_1_2/1(q) -> cmult0_2_1/3
  c_to_ri2_1/2 -> pipeline0_2_1_3/1(d), pipeline1_1_1_3/1(d), pipeline2_0_1_1/1(d), pipeline2_0_1_3/1(d), pipeline2_1_1_1/1(d)
  pipeline0_2_1_3/1(q) -> cmult0_2_1/4
  cmult0_2_1/1 -> cvrt0_2_1_real/1(din)
  cmult0_2_1/2 -> cvrt0_2_1_imag/1(din)
  cvrt0_2_1_real/1(dout) -> ri_to_c0_2_1/1
  cvrt0_2_1_imag/1(dout) -> ri_to_c0_2_1/2
  ri_to_c0_2_1/1 -> pack0_2/2(in2)
  pack0_2/1(bus_out) -> din0_x_din2*/1
  pipeline0_3_0_0/1(q) -> cmult0_3_0/1
  pipeline0_3_0_1/1(q) -> cmult0_3_0/2
  c_to_ri3_0/1 -> pipeline0_3_0_2/1(d), pipeline1_2_0_2/1(d), pipeline2_1_0_2/1(d), pipeline3_0_0_0/1(d), pipeline3_0_0_2/1(d)
  pipeline0_3_0_2/1(q) -> cmult0_3_0/3
  c_to_ri3_0/2 -> pipeline0_3_0_3/1(d), pipeline1_2_0_3/1(d), pipeline2_1_0_3/1(d), pipeline3_0_0_1/1(d), pipeline3_0_0_3/1(d)
  pipeline0_3_0_3/1(q) -> cmult0_3_0/4
  cmult0_3_0/1 -> cvrt0_3_0_real/1(din)
  cmult0_3_0/2 -> cvrt0_3_0_imag/1(din)
  cvrt0_3_0_real/1(dout) -> ri_to_c0_3_0/1
  cvrt0_3_0_imag/1(dout) -> ri_to_c0_3_0/2
  ri_to_c0_3_0/1 -> pack0_3/1(in1)
  pipeline0_3_1_0/1(q) -> cmult0_3_1/1
  pipeline0_3_1_1/1(q) -> cmult0_3_1/2
  c_to_ri3_1/1 -> pipeline0_3_1_2/1(d), pipeline1_2_1_2/1(d), pipeline2_1_1_2/1(d), pipeline3_0_1_0/1(d), pipeline3_0_1_2/1(d)
  pipeline0_3_1_2/1(q) -> cmult0_3_1/3
  c_to_ri3_1/2 -> pipeline0_3_1_3/1(d), pipeline1_2_1_3/1(d), pipeline2_1_1_3/1(d), pipeline3_0_1_1/1(d), pipeline3_0_1_3/1(d)
  pipeline0_3_1_3/1(q) -> cmult0_3_1/4
  cmult0_3_1/1 -> cvrt0_3_1_real/1(din)
  cmult0_3_1/2 -> cvrt0_3_1_imag/1(din)
  cvrt0_3_1_real/1(dout) -> ri_to_c0_3_1/1
  cvrt0_3_1_imag/1(dout) -> ri_to_c0_3_1/2
  ri_to_c0_3_1/1 -> pack0_3/2(in2)
  pack0_3/1(bus_out) -> din0_x_din3*/1
  pipeline1_0_0_0/1(q) -> cmult1_0_0/1
  pipeline1_0_0_1/1(q) -> cmult1_0_0/2
  pipeline1_0_0_2/1(q) -> cmult1_0_0/3
  pipeline1_0_0_3/1(q) -> cmult1_0_0/4
  cmult1_0_0/1 -> cvrt1_0_0_real/1(din)
  cmult1_0_0/2 -> cvrt1_0_0_imag/1(din)
  cvrt1_0_0_real/1(dout) -> ri_to_c1_0_0/1
  cvrt1_0_0_imag/1(dout) -> ri_to_c1_0_0/2
  ri_to_c1_0_0/1 -> pack1_0/1(in1)
  pipeline1_0_1_0/1(q) -> cmult1_0_1/1
  pipeline1_0_1_1/1(q) -> cmult1_0_1/2
  pipeline1_0_1_2/1(q) -> cmult1_0_1/3
  pipeline1_0_1_3/1(q) -> cmult1_0_1/4
  cmult1_0_1/1 -> cvrt1_0_1_real/1(din)
  cmult1_0_1/2 -> cvrt1_0_1_imag/1(din)
  cvrt1_0_1_real/1(dout) -> ri_to_c1_0_1/1
  cvrt1_0_1_imag/1(dout) -> ri_to_c1_0_1/2
  ri_to_c1_0_1/1 -> pack1_0/2(in2)
  pack1_0/1(bus_out) -> din1_x_din1*/1
  pipeline1_1_0_0/1(q) -> cmult1_1_0/1
  pipeline1_1_0_1/1(q) -> cmult1_1_0/2
  pipeline1_1_0_2/1(q) -> cmult1_1_0/3
  pipeline1_1_0_3/1(q) -> cmult1_1_0/4
  cmult1_1_0/1 -> cvrt1_1_0_real/1(din)
  cmult1_1_0/2 -> cvrt1_1_0_imag/1(din)
  cvrt1_1_0_real/1(dout) -> ri_to_c1_1_0/1
  cvrt1_1_0_imag/1(dout) -> ri_to_c1_1_0/2
  ri_to_c1_1_0/1 -> pack1_1/1(in1)
  pipeline1_1_1_0/1(q) -> cmult1_1_1/1
  pipeline1_1_1_1/1(q) -> cmult1_1_1/2
  pipeline1_1_1_2/1(q) -> cmult1_1_1/3
  pipeline1_1_1_3/1(q) -> cmult1_1_1/4
  cmult1_1_1/1 -> cvrt1_1_1_real/1(din)
  cmult1_1_1/2 -> cvrt1_1_1_imag/1(din)
  cvrt1_1_1_real/1(dout) -> ri_to_c1_1_1/1
  cvrt1_1_1_imag/1(dout) -> ri_to_c1_1_1/2
  ri_to_c1_1_1/1 -> pack1_1/2(in2)
  pack1_1/1(bus_out) -> din1_x_din2*/1
  pipeline1_2_0_0/1(q) -> cmult1_2_0/1
  pipeline1_2_0_1/1(q) -> cmult1_2_0/2
  pipeline1_2_0_2/1(q) -> cmult1_2_0/3
  pipeline1_2_0_3/1(q) -> cmult1_2_0/4
  cmult1_2_0/1 -> cvrt1_2_0_real/1(din)
  cmult1_2_0/2 -> cvrt1_2_0_imag/1(din)
  cvrt1_2_0_real/1(dout) -> ri_to_c1_2_0/1
  cvrt1_2_0_imag/1(dout) -> ri_to_c1_2_0/2
  ri_to_c1_2_0/1 -> pack1_2/1(in1)
  pipeline1_2_1_0/1(q) -> cmult1_2_1/1
  pipeline1_2_1_1/1(q) -> cmult1_2_1/2
  pipeline1_2_1_2/1(q) -> cmult1_2_1/3
  pipeline1_2_1_3/1(q) -> cmult1_2_1/4
  cmult1_2_1/1 -> cvrt1_2_1_real/1(din)
  cmult1_2_1/2 -> cvrt1_2_1_imag/1(din)
  cvrt1_2_1_real/1(dout) -> ri_to_c1_2_1/1
  cvrt1_2_1_imag/1(dout) -> ri_to_c1_2_1/2
  ri_to_c1_2_1/1 -> pack1_2/2(in2)
  pack1_2/1(bus_out) -> din1_x_din3*/1
  pipeline2_0_0_0/1(q) -> cmult2_0_0/1
  pipeline2_0_0_1/1(q) -> cmult2_0_0/2
  pipeline2_0_0_2/1(q) -> cmult2_0_0/3
  pipeline2_0_0_3/1(q) -> cmult2_0_0/4
  cmult2_0_0/1 -> cvrt2_0_0_real/1(din)
  cmult2_0_0/2 -> cvrt2_0_0_imag/1(din)
  cvrt2_0_0_real/1(dout) -> ri_to_c2_0_0/1
  cvrt2_0_0_imag/1(dout) -> ri_to_c2_0_0/2
  ri_to_c2_0_0/1 -> pack2_0/1(in1)
  pipeline2_0_1_0/1(q) -> cmult2_0_1/1
  pipeline2_0_1_1/1(q) -> cmult2_0_1/2
  pipeline2_0_1_2/1(q) -> cmult2_0_1/3
  pipeline2_0_1_3/1(q) -> cmult2_0_1/4
  cmult2_0_1/1 -> cvrt2_0_1_real/1(din)
  cmult2_0_1/2 -> cvrt2_0_1_imag/1(din)
  cvrt2_0_1_real/1(dout) -> ri_to_c2_0_1/1
  cvrt2_0_1_imag/1(dout) -> ri_to_c2_0_1/2
  ri_to_c2_0_1/1 -> pack2_0/2(in2)
  pack2_0/1(bus_out) -> din2_x_din2*/1
  pipeline2_1_0_0/1(q) -> cmult2_1_0/1
  pipeline2_1_0_1/1(q) -> cmult2_1_0/2
  pipeline2_1_0_2/1(q) -> cmult2_1_0/3
  pipeline2_1_0_3/1(q) -> cmult2_1_0/4
  cmult2_1_0/1 -> cvrt2_1_0_real/1(din)
  cmult2_1_0/2 -> cvrt2_1_0_imag/1(din)
  cvrt2_1_0_real/1(dout) -> ri_to_c2_1_0/1
  cvrt2_1_0_imag/1(dout) -> ri_to_c2_1_0/2
  ri_to_c2_1_0/1 -> pack2_1/1(in1)
  pipeline2_1_1_0/1(q) -> cmult2_1_1/1
  pipeline2_1_1_1/1(q) -> cmult2_1_1/2
  pipeline2_1_1_2/1(q) -> cmult2_1_1/3
  pipeline2_1_1_3/1(q) -> cmult2_1_1/4
  cmult2_1_1/1 -> cvrt2_1_1_real/1(din)
  cmult2_1_1/2 -> cvrt2_1_1_imag/1(din)
  cvrt2_1_1_real/1(dout) -> ri_to_c2_1_1/1
  cvrt2_1_1_imag/1(dout) -> ri_to_c2_1_1/2
  ri_to_c2_1_1/1 -> pack2_1/2(in2)
  pack2_1/1(bus_out) -> din2_x_din3*/1
  pipeline3_0_0_0/1(q) -> cmult3_0_0/1
  pipeline3_0_0_1/1(q) -> cmult3_0_0/2
  pipeline3_0_0_2/1(q) -> cmult3_0_0/3
  pipeline3_0_0_3/1(q) -> cmult3_0_0/4
  cmult3_0_0/1 -> cvrt3_0_0_real/1(din)
  cmult3_0_0/2 -> cvrt3_0_0_imag/1(din)
  cvrt3_0_0_real/1(dout) -> ri_to_c3_0_0/1
  cvrt3_0_0_imag/1(dout) -> ri_to_c3_0_0/2
  ri_to_c3_0_0/1 -> pack3_0/1(in1)
  pipeline3_0_1_0/1(q) -> cmult3_0_1/1
  pipeline3_0_1_1/1(q) -> cmult3_0_1/2
  pipeline3_0_1_2/1(q) -> cmult3_0_1/3
  pipeline3_0_1_3/1(q) -> cmult3_0_1/4
  cmult3_0_1/1 -> cvrt3_0_1_real/1(din)
  cmult3_0_1/2 -> cvrt3_0_1_imag/1(din)
  cvrt3_0_1_real/1(dout) -> ri_to_c3_0_1/1
  cvrt3_0_1_imag/1(dout) -> ri_to_c3_0_1/2
  ri_to_c3_0_1/1 -> pack3_0/2(in2)
  pack3_0/1(bus_out) -> din3_x_din3*/1
